simulink library "Renault_Library"  (Simulink 8.2) (continued)
== system Renault_Library/Services ECU/Diag == (continued)
  RSA_DiagnosticManagement_v02.00 : Subsystem  Tag=[LIB]  mask(Diagnostic){DiagnosticSessionControl=on, DefaultSession=Activated, ProgrammingSession=Activated, ExtendedDiagnosticSession=Activated, SafetySystemDiagnosticSession=Activated, EcuReset=off, EcuResetSession1=off, EcuResetSession2=off, EcuResetSession3=off, EcuResetSession4=off, HardReset=off, HardResetSession1=off, HardResetSession2=off, HardResetSession3=off, HardResetSession4=off, KeyOffReset=off, KeyOffResetSession1=off, KeyOffResetSession2=off, KeyOffResetSession3=off, KeyOffResetSession4=off, SoftReset=off, SoftResetSession1=off, SoftResetSession2=off, SoftResetSession3=off, SoftResetSession4=off, EnableRapidPowerShutDown=off, EnableRapidPowerShutDownSession1=off, EnableRapidPowerShutDownSession2=off, EnableRapidPowerShutDownSession3=off, EnableRapidPowerShutDownSession4=off, DisableRapidPowerShutDown=off, DisableRapidPowerShutDownSession1=off, DisableRapidPowerShutDownSession2=off, DisableRapidPowerShutDownSession3=off, DisableRapidPowerShutDownSession4=off, SecurityAccess=off, SecurityAccessSession1=off, SecurityAccessSession2=off, SecurityAccessSession3=off, SecurityAccessSession4=off, RequestSeed=off, RequestSeedSession1=off, RequestSeedSession2=off, RequestSeedSession3=off, RequestSeedSession4=off, SendKey=off, SendKeySession1=off, SendKeySession2=off, SendKeySession3=off, SendKeySession4=off, RequestSeed3=off, RequestSeed3Session1=off, RequestSeed3Session2=off, RequestSeed3Session3=off, RequestSeed3Session4=off, RequestSeed5=off, RequestSeed5Session1=off, RequestSeed5Session2=off, RequestSeed5Session3=off, RequestSeed5Session4=off, RequestSeed7=off, RequestSeed7Session1=off, RequestSeed7Session2=off, RequestSeed7Session3=off, RequestSeed7Session4=off, RequestSeed41=off, RequestSeed41Session1=off, RequestSeed41Session2=off, RequestSeed41Session3=off, RequestSeed41Session4=off, SendKey4=off, SendKey4Session1=off, SendKey4Session2=off, SendKey4Session3=off, SendKey4Session4=off, SendKey6=off, SendKey6Session1=off, SendKey6Session2=off, SendKey6Session3=off, SendKey6Session4=off, SendKey8=off, SendKey8Session1=off, SendKey8Session2=off, SendKey8Session3=off, SendKey8Session4=off, SendKey42=off, SendKey42Session1=off, SendKey42Session2=off, SendKey42Session3=off, SendKey42Session4=off, CommunicationControl=off, CommunicationControlSession1=off, CommunicationControlSession2=off, CommunicationControlSession3=off, CommunicationControlSession4=off, EnableRxAndTx=off, EnableRxAndTxSession1=off, EnableRxAndTxSession2=off, EnableRxAndTxSession3=off, EnableRxAndTxSession4=off, EnableRxAndDisableTx=off, EnableRxAndDisableTxSession1=off, EnableRxAndDisableTxSession2=off, EnableRxAndDisableTxSession3=off, EnableRxAndDisableTxSession4=off, DisableRxAndEnableTx=off, DisableRxAndEnableTxSession1=off, DisableRxAndEnableTxSession2=off, DisableRxAndEnableTxSession3=off, DisableRxAndEnableTxSession4=off, DisableRxAndTx=off, DisableRxAndTxSession1=off, DisableRxAndTxSession2=off, DisableRxAndTxSession3=off, DisableRxAndTxSession4=off, EnableRxAndDisableTxWithEnhanceAddressInformation=off, EnableRxAndDisableTxWithEnhanceAddressInformationSession1=off, EnableRxAndDisableTxWithEnhanceAddressInformationSession2=off, EnableRxAndDisableTxWithEnhanceAddressInformationSession3=off, EnableRxAndDisableTxWithEnhanceAddressInformationSession4=off, EnableRxAndTxWithEnhanceAddressInformation=off, EnableRxAndTxWithEnhanceAddressInformationSession1=off, EnableRxAndTxWithEnhanceAddressInformationSession2=off, EnableRxAndTxWithEnhanceAddressInformationSession3=off, EnableRxAndTxWithEnhanceAddressInformationSession4=off, TesterPresent=on, TesterPresentSession1=on, TesterPresentSession2=on, TesterPresentSession3=on, TesterPresentSession4=on, ZeroSubFunction=on, ZeroSubFunctionSession1=on, ZeroSubFunctionSession2=on, ZeroSubFunctionSession3=on, ZeroSubFunctionSession4=on, ZeroSubFunctionWithoutResponse=on, ZeroSubFunctionWithoutResponseSession1=on, ZeroSubFunctionWithoutResponseSession2=on, ZeroSubFunctionWithoutResponseSession3=on, ZeroSubFunctionWithoutResponseSession4=on, AccessTimingParameter=off, AccessTimingParameterSession1=off, AccessTimingParameterSession2=off, AccessTimingParameterSession3=off, AccessTimingParameterSession4=off, ReadExtendedTimingParamaterSet=off, ReadExtendedTimingParamaterSetSession1=off, ReadExtendedTimingParamaterSetSession2=off, ReadExtendedTimingParamaterSetSession3=off, ReadExtendedTimingParamaterSetSession4=off, SetTimmingParametersToDefaultValues=off, SetTimmingParametersToDefaultValuesSession1=off, SetTimmingParametersToDefaultValuesSession2=off, SetTimmingParametersToDefaultValuesSession3=off, SetTimmingParametersToDefaultValuesSession4=off, ReadCurrentActiveTimmingParamaters=off, ReadCurrentActiveTimmingParamatersSession1=off, ReadCurrentActiveTimmingParamatersSession2=off, ReadCurrentActiveTimmingParamatersSession3=off, ReadCurrentActiveTimmingParamatersSession4=off, SetTimmingParametersToGivenValues=off, SetTimmingParametersToGivenValuesSession1=off, SetTimmingParametersToGivenValuesSession2=off, SetTimmingParametersToGivenValuesSession3=off, SetTimmingParametersToGivenValuesSession4=off, SecuredDataTransmission=off, SecuredDataTransmissionSession1=off, SecuredDataTransmissionSession2=off, SecuredDataTransmissionSession3=off, SecuredDataTransmissionSession4=off, ControlDTCSetting=off, ControlDTCSettingSession1=off, ControlDTCSettingSession2=off, ControlDTCSettingSession3=off, ControlDTCSettingSession4=off, On=off, OnSession1=off, OnSession2=off, OnSession3=off, OnSession4=off, Off=off, OffSession1=off, OffSession2=off, OffSession3=off, OffSession4=off, ResponseOnEvent=off, ResponseOnEventSession1=off, ResponseOnEventSession2=off, ResponseOnEventSession3=off, ResponseOnEventSession4=off, StopResponseOnEvent=off, StopResponseOnEventSession1=off, StopResponseOnEventSession2=off, StopResponseOnEventSession3=off, StopResponseOnEventSession4=off, OnDTCStatusChange=off, OnDTCStatusChangeSession1=off, OnDTCStatusChangeSession2=off, OnDTCStatusChangeSession3=off, OnDTCStatusChangeSession4=off, OnTimeInterrupt=off, OnTimeInterruptSession1=off, OnTimeInterruptSession2=off, OnTimeInterruptSession3=off, OnTimeInterruptSession4=off, OnChangeOfDataIdentifier=off, OnChangeOfDataIdentifierSession1=off, OnChangeOfDataIdentifierSession2=off, OnChangeOfDataIdentifierSession3=off, OnChangeOfDataIdentifierSession4=off, ReportActivedEvents=off, ReportActivedEventsSession1=off, ReportActivedEventsSession2=off, ReportActivedEventsSession3=off, ReportActivedEventsSession4=off, StartResponseOnEvent=off, StartResponseOnEventSession1=off, StartResponseOnEventSession2=off, StartResponseOnEventSession3=off, StartResponseOnEventSession4=off, ClearResponseOnEvent=off, ClearResponseOnEventSession1=off, ClearResponseOnEventSession2=off, ClearResponseOnEventSession3=off, ClearResponseOnEventSession4=off, OnComparisonOfValues=off, OnComparisonOfValuesSession1=off, OnComparisonOfValuesSession2=off, OnComparisonOfValuesSession3=off, OnComparisonOfValuesSession4=off, LinControl=off, LinControlSession1=off, LinControlSession2=off, LinControlSession3=off, LinControlSession4=off, VerifyModeTransitionWithFixedParamater=off, VerifyModeTransitionWithFixedParamaterSession1=off, VerifyModeTransitionWithFixedParamaterSession2=off, VerifyModeTransitionWithFixedParamaterSession3=off, VerifyModeTransitionWithFixedParamaterSession4=off, VerifyModeTransitionWithSpecificParamater=off, VerifyModeTransitionWithSpecificParamaterSession1=off, VerifyModeTransitionWithSpecificParamaterSession2=off, VerifyModeTransitionWithSpecificParamaterSession3=off, VerifyModeTransitionWithSpecificParamaterSession4=off, TransitionMode=off, TransitionModeSession1=off, TransitionModeSession2=off, TransitionModeSession3=off, TransitionModeSession4=off, ReadDataByIdentifier=on, ReadDataByIdentifierSession1=on, ReadDataByIdentifierSession2=on, ReadDataByIdentifierSession3=on, ReadDataByIdentifierSession4=on, ReadMemoryByAddress=on, ReadMemoryByAddressSession1=on, ReadMemoryByAddressSession2=off, ReadMemoryByAddressSession3=off, ReadMemoryByAddressSession4=off, ReadScalingDataByIdentifier=off, ReadScalingDataByIdentifierSession1=off, ReadScalingDataByIdentifierSession2=off, ReadScalingDataByIdentifierSession3=off, ReadScalingDataByIdentifierSession4=off, ReadDataByPeriodicIdentifier=off, ReadDataByPeriodicIdentifierSession1=off, ReadDataByPeriodicIdentifierSession2=off, ReadDataByPeriodicIdentifierSession3=off, ReadDataByPeriodicIdentifierSession4=off, DynamicallyDefineDataIdentifier=off, DynamicallyDefineDataIdentifierSession1=off, DynamicallyDefineDataIdentifierSession2=off, DynamicallyDefineDataIdentifierSession3=off, DynamicallyDefineDataIdentifierSession4=off, DefineByIdentifier=off, DefineByIdentifierSession1=off, DefineByIdentifierSession2=off, DefineByIdentifierSession3=off, DefineByIdentifierSession4=off, DefineByMemoryAddress=off, DefineByMemoryAddressSession1=off, DefineByMemoryAddressSession2=off, DefineByMemoryAddressSession3=off, DefineByMemoryAddressSession4=off, ClearDynamicallyDefinedDataIdentifier=off, ClearDynamicallyDefinedDataIdentifierSession1=off, ClearDynamicallyDefinedDataIdentifierSession2=off, ClearDynamicallyDefinedDataIdentifierSession3=off, ClearDynamicallyDefinedDataIdentifierSession4=off, WriteDataByIdentifier=on, WriteDataByIdentifierSession1=on, WriteDataByIdentifierSession2=on, WriteDataByIdentifierSession3=on, WriteDataByIdentifierSession4=on, WriteDataByAddress=off, WriteDataByAddressSession1=on, WriteDataByAddressSession2=off, WriteDataByAddressSession3=off, WriteDataByAddressSession4=off, ClearDiagnosticInformation=on, ClearDiagnosticInformationSession1=on, ClearDiagnosticInformationSession2=on, ClearDiagnosticInformationSession3=on, ClearDiagnosticInformationSession4=on, ReadDTCInformation=on, ReadDTCInformationSession1=on, ReadDTCInformationSession2=on, ReadDTCInformationSession3=on, ReadDTCInformationSession4=on, ReportNumberOfDTCByStatusMask=on, ReportNumberOfDTCByStatusMaskSession1=on, ReportNumberOfDTCByStatusMaskSession2=on, ReportNumberOfDTCByStatusMaskSession3=on, ReportNumberOfDTCByStatusMaskSession4=on, ReportDTCByStatusMask=on, ReportDTCByStatusMaskSession1=on, ReportDTCByStatusMaskSession2=on, ReportDTCByStatusMaskSession3=on, ReportDTCByStatusMaskSession4=on, ReporDTCSnapshotIdentification=on, ReporDTCSnapshotIdentificationSession1=on, ReporDTCSnapshotIdentificationSession2=on, ReporDTCSnapshotIdentificationSession3=on, ReporDTCSnapshotIdentificationSession4=on, ReporDTCSnapshotRecordByDTCNumber=off, ReporDTCSnapshotRecordByDTCNumberSession1=off, ReporDTCSnapshotRecordByDTCNumberSession2=off, ReporDTCSnapshotRecordByDTCNumberSession3=off, ReporDTCSnapshotRecordByDTCNumberSession4=off, ReportDTCStoredDataByRecordNumber=off, ReportDTCStoredDataByRecordNumberSession1=off, ReportDTCStoredDataByRecordNumberSession2=off, ReportDTCStoredDataByRecordNumberSession3=off, ReportDTCStoredDataByRecordNumberSession4=off, ReportDTCExtendedDataRecordByDTCNumber=off, ReportDTCExtendedDataRecordByDTCNumberSession1=off, ReportDTCExtendedDataRecordByDTCNumberSession2=off, ReportDTCExtendedDataRecordByDTCNumberSession3=off, ReportDTCExtendedDataRecordByDTCNumberSession4=off, ReportNumberOfDTCBySeverityMaskRecord=off, ReportNumberOfDTCBySeverityMaskRecordSession1=off, ReportNumberOfDTCBySeverityMaskRecordSession2=off, ReportNumberOfDTCBySeverityMaskRecordSession3=off, ReportNumberOfDTCBySeverityMaskRecordSession4=off, ReportDTCBySeverityMaskRecord=off, ReportDTCBySeverityMaskRecordSession1=off, ReportDTCBySeverityMaskRecordSession2=off, ReportDTCBySeverityMaskRecordSession3=off, ReportDTCBySeverityMaskRecordSession4=off, ReportSeverityInformationOfDTC=off, ReportSeverityInformationOfDTCSession1=off, ReportSeverityInformationOfDTCSession2=off, ReportSeverityInformationOfDTCSession3=off, ReportSeverityInformationOfDTCSession4=off, ReportSupportedDTC=on, ReportSupportedDTCSession1=on, ReportSupportedDTCSession2=on, ReportSupportedDTCSession3=on, ReportSupportedDTCSession4=on, ReportFirstTestFailedDTC=off, ReportFirstTestFailedDTCSession1=off, ReportFirstTestFailedDTCSession2=off, ReportFirstTestFailedDTCSession3=off, ReportFirstTestFailedDTCSession4=off, ReportFirstConfirmedDTC=off, ReportFirstConfirmedDTCSession1=off, ReportFirstConfirmedDTCSession2=off, ReportFirstConfirmedDTCSession3=off, ReportFirstConfirmedDTCSession4=off, ReportMostRecentTestFailedDTC=off, ReportMostRecentTestFailedDTCSession1=off, ReportMostRecentTestFailedDTCSession2=off, ReportMostRecentTestFailedDTCSession3=off, ReportMostRecentTestFailedDTCSession4=off, ReportMostRecentConfimedDTC=off, ReportMostRecentConfimedDTCSession1=off, ReportMostRecentConfimedDTCSession2=off, ReportMostRecentConfimedDTCSession3=off, ReportMostRecentConfimedDTCSession4=off, ReportMirrorMemoryDTCByStatusMask=off, ReportMirrorMemoryDTCByStatusMaskSession1=off, ReportMirrorMemoryDTCByStatusMaskSession2=off, ReportMirrorMemoryDTCByStatusMaskSession3=off, ReportMirrorMemoryDTCByStatusMaskSession4=off, ReportMirrorMemoryDTCExtendedDataRecordByDTCNumber=off, ReportMirrorMemoryDTCExtendedDataRecordByDTCNumberSession1=off, ReportMirrorMemoryDTCExtendedDataRecordByDTCNumberSession2=off, ReportMirrorMemoryDTCExtendedDataRecordByDTCNumberSession3=off, ReportMirrorMemoryDTCExtendedDataRecordByDTCNumberSession4=off, ReportNumberOfMirrorMemoryDTCByStatusMask=off, ReportNumberOfMirrorMemoryDTCByStatusMaskSession1=off, ReportNumberOfMirrorMemoryDTCByStatusMaskSession2=off, ReportNumberOfMirrorMemoryDTCByStatusMaskSession3=off, ReportNumberOfMirrorMemoryDTCByStatusMaskSession4=off, ReportNumberOfEmissionsRelatedOBDDTCByStatusMask=off, ReportNumberOfEmissionsRelatedOBDDTCByStatusMaskSession1=off, ReportNumberOfEmissionsRelatedOBDDTCByStatusMaskSession2=off, ReportNumberOfEmissionsRelatedOBDDTCByStatusMaskSession3=off, ReportNumberOfEmissionsRelatedOBDDTCByStatusMaskSession4=off, ReportEmissionsRelatedOBDDTCByStatusMask=off, ReportEmissionsRelatedOBDDTCByStatusMaskSession1=off, ReportEmissionsRelatedOBDDTCByStatusMaskSession2=off, ReportEmissionsRelatedOBDDTCByStatusMaskSession3=off, ReportEmissionsRelatedOBDDTCByStatusMaskSession4=off, ReportDTCFaultDetectionCounter=off, ReportDTCFaultDetectionCounterSession1=off, ReportDTCFaultDetectionCounterSession2=off, ReportDTCFaultDetectionCounterSession3=off, ReportDTCFaultDetectionCounterSession4=off, ReportDTCWithPermanetStatus=off, ReportDTCWithPermanetStatusSession1=off, ReportDTCWithPermanetStatusSession2=off, ReportDTCWithPermanetStatusSession3=off, ReportDTCWithPermanetStatusSession4=off, ReportWWHOBDDTCByMaskRecord=off, ReportWWHOBDDTCByMaskRecordSession1=off, ReportWWHOBDDTCByMaskRecordSession2=off, ReportWWHOBDDTCByMaskRecordSession3=off, ReportWWHOBDDTCByMaskRecordSession4=off, ReportWWHOBDDTCWithPermanentStatus=off, ReportWWHOBDDTCWithPermanentStatusSession1=off, ReportWWHOBDDTCWithPermanentStatusSession2=off, ReportWWHOBDDTCWithPermanentStatusSession3=off, ReportWWHOBDDTCWithPermanentStatusSession4=off, InputOutputConrolByIdentifier=on, InputOutputConrolByIdentifierSession1=on, InputOutputConrolByIdentifierSession2=on, InputOutputConrolByIdentifierSession3=on, InputOutputConrolByIdentifierSession4=on, RoutineControl=on, RoutineControlSession1=on, RoutineControlSession2=off, RoutineControlSession3=on, RoutineControlSession4=on, StartRoutine=on, StartRoutineSession1=on, StartRoutineSession2=on, StartRoutineSession3=on, StartRoutineSession4=on, StopRoutine=on, StopRoutineSession1=on, StopRoutineSession2=on, StopRoutineSession3=on, StopRoutineSession4=on, RequestRoutineResults=off, RequestRoutineResultsSession1=off, RequestRoutineResultsSession2=off, RequestRoutineResultsSession3=off, RequestRoutineResultsSession4=off, RequestDownload=off, RequestDownloadSession1=off, RequestDownloadSession2=off, RequestDownloadSession3=off, RequestDownloadSession4=off, RequestUpload=off, RequestUploadSession1=off, RequestUploadSession2=off, RequestUploadSession3=off, RequestUploadSession4=off, TransfertData=off, TransfertDataSession1=off, TransfertDataSession2=off, TransfertDataSession3=off, TransfertDataSession4=off, RequestTransfertExit=off, RequestTransfertExitSession1=off, RequestTransfertExitSession2=off, RequestTransfertExitSession3=off, RequestTransfertExitSession4=off}
      mask prompts: DiagnosticSessionControl "DiagnosticSessionControl (0x10)"; DefaultSession "DefaultSession (0x01)"; ProgrammingSession "ProgrammingSession (0x02)"; ExtendedDiagnosticSession "ExtendedDiagnosticSession (0x03)"; SafetySystemDiagnosticSession "SafetySystemDiagnosticSession (0x04)"; EcuReset "EcuReset (0x11)"; EcuResetSession1 "EcuReset (0x11)"; EcuResetSession2 "EcuReset (0x11)"; EcuResetSession3 "EcuReset (0x11)"; EcuResetSession4 "EcuReset (0x11)"; HardReset "HardReset (0x01)"; HardResetSession1 "HardReset (0x01)"; HardResetSession2 "HardReset (0x01)"; HardResetSession3 "HardReset (0x01)"; HardResetSession4 "HardReset (0x01)"; KeyOffReset "KeyOffReset (0x02)"; KeyOffResetSession1 "KeyOffReset (0x02)"; KeyOffResetSession2 "KeyOffReset (0x02)"; KeyOffResetSession3 "KeyOffReset (0x02)"; KeyOffResetSession4 "KeyOffReset (0x02)"; SoftReset "SoftReset (0x03)"; SoftResetSession1 "SoftReset (0x03)"; SoftResetSession2 "SoftReset (0x03)"; SoftResetSession3 "SoftReset (0x03)"; SoftResetSession4 "SoftReset (0x03)"; EnableRapidPowerShutDown "EnableRapidPowerShutDown (0x04)"; EnableRapidPowerShutDownSession1 "EnableRapidPowerShutDown (0x04)"; EnableRapidPowerShutDownSession2 "EnableRapidPowerShutDown (0x04)"; EnableRapidPowerShutDownSession3 "EnableRapidPowerShutDown (0x04)"; EnableRapidPowerShutDownSession4 "EnableRapidPowerShutDown (0x04)"; DisableRapidPowerShutDown "DisableRapidPowerShutDown (0x05)"; DisableRapidPowerShutDownSession1 "DisableRapidPowerShutDown (0x05)"; DisableRapidPowerShutDownSession2 "DisableRapidPowerShutDown (0x05)"; DisableRapidPowerShutDownSession3 "DisableRapidPowerShutDown (0x05)"; DisableRapidPowerShutDownSession4 "DisableRapidPowerShutDown (0x05)"; SecurityAccess "SecurityAccess (0x27)"; SecurityAccessSession1 "SecurityAccess (0x27)"; SecurityAccessSession2 "SecurityAccess (0x27)"; SecurityAccessSession3 "SecurityAccess (0x27)"; SecurityAccessSession4 "SecurityAccess (0x27)"; RequestSeed "RequestSeed (0x01)"; RequestSeedSession1 "RequestSeed (0x01)"; RequestSeedSession2 "RequestSeed (0x01)"; RequestSeedSession3 "RequestSeed (0x01)"; RequestSeedSession4 "RequestSeed (0x01)"; SendKey "SendKey (0x02)"; SendKeySession1 "SendKey (0x02)"; SendKeySession2 "SendKey (0x02)"; SendKeySession3 "SendKey (0x02)"; SendKeySession4 "SendKey (0x02)"; RequestSeed3 "RequestSeed (0x03)"; RequestSeed3Session1 "RequestSeed (0x03)"; RequestSeed3Session2 "RequestSeed (0x03)"; RequestSeed3Session3 "RequestSeed (0x03)"; RequestSeed3Session4 "RequestSeed (0x03)"; RequestSeed5 "RequestSeed (0x05)"; RequestSeed5Session1 "RequestSeed (0x05)"; RequestSeed5Session2 "RequestSeed (0x05)"; RequestSeed5Session3 "RequestSeed (0x05)"; RequestSeed5Session4 "RequestSeed (0x05)"; RequestSeed7 "RequestSeed (0x07)"; RequestSeed7Session1 "RequestSeed (0x07)"; RequestSeed7Session2 "RequestSeed (0x07)"; RequestSeed7Session3 "RequestSeed (0x07)"; RequestSeed7Session4 "RequestSeed (0x07)"; RequestSeed41 "RequestSeed (0x41)"; RequestSeed41Session1 "RequestSeed (0x41)"; RequestSeed41Session2 "RequestSeed (0x41)"; RequestSeed41Session3 "RequestSeed (0x41)"; RequestSeed41Session4 "RequestSeed (0x41)"; SendKey4 "SendKey (0x04)"; SendKey4Session1 "SendKey (0x04)"; SendKey4Session2 "SendKey (0x04)"; SendKey4Session3 "SendKey (0x04)"; SendKey4Session4 "SendKey (0x04)"; SendKey6 "SendKey (0x06)"; SendKey6Session1 "SendKey (0x06)"; SendKey6Session2 "SendKey (0x06)"; SendKey6Session3 "SendKey (0x06)"; SendKey6Session4 "SendKey (0x06)"; SendKey8 "SendKey (0x08)"; SendKey8Session1 "SendKey (0x08)"; SendKey8Session2 "SendKey (0x08)"; SendKey8Session3 "SendKey (0x08)"; SendKey8Session4 "SendKey (0x08)"; SendKey42 "SendKey (0x42)"; SendKey42Session1 "SendKey (0x42)"; SendKey42Session2 "SendKey (0x42)"; SendKey42Session3 "SendKey (0x42)"; SendKey42Session4 "SendKey (0x42)"; CommunicationControl "CommunicationControl (0x28)"; CommunicationControlSession1 "CommunicationControl (0x28)"; CommunicationControlSession2 "CommunicationControl (0x28)"; CommunicationControlSession3 "CommunicationControl (0x28)"; CommunicationControlSession4 "CommunicationControl (0x28)"; EnableRxAndTx "EnableRxAndTx (0x00)"; EnableRxAndTxSession1 "EnableRxAndTx (0x00)"; EnableRxAndTxSession2 "EnableRxAndTx (0x00)"; EnableRxAndTxSession3 "EnableRxAndTx (0x00)"; EnableRxAndTxSession4 "EnableRxAndTx (0x00)"; EnableRxAndDisableTx "EnableRxAndDisableTx (0x01)"; EnableRxAndDisableTxSession1 "EnableRxAndDisableTx (0x01)"; EnableRxAndDisableTxSession2 "EnableRxAndDisableTx (0x01)"; EnableRxAndDisableTxSession3 "EnableRxAndDisableTx (0x01)"; EnableRxAndDisableTxSession4 "EnableRxAndDisableTx (0x01)"; DisableRxAndEnableTx "DisableRxAndEnableTx (0x02)"; DisableRxAndEnableTxSession1 "DisableRxAndEnableTx (0x02)"; DisableRxAndEnableTxSession2 "DisableRxAndEnableTx (0x02)"; DisableRxAndEnableTxSession3 "DisableRxAndEnableTx (0x02)"; DisableRxAndEnableTxSession4 "DisableRxAndEnableTx (0x02)"; DisableRxAndTx "DisableRxAndTx (0x03)"; DisableRxAndTxSession1 "DisableRxAndTx (0x03)"; DisableRxAndTxSession2 "DisableRxAndTx (0x03)"; DisableRxAndTxSession3 "DisableRxAndTx (0x03)"; DisableRxAndTxSession4 "DisableRxAndTx (0x03)"; EnableRxAndDisableTxWithEnhanceAddressInformation "EnableRxAndDisableTxWithEnhanceAddressInformation (0x04)"; EnableRxAndDisableTxWithEnhanceAddressInformationSession1 "EnableRxAndDisableTxWithEnhanceAddressInformation (0x04)"; EnableRxAndDisableTxWithEnhanceAddressInformationSession2 "EnableRxAndDisableTxWithEnhanceAddressInformation (0x04)"; EnableRxAndDisableTxWithEnhanceAddressInformationSession3 "EnableRxAndDisableTxWithEnhanceAddressInformation (0x04)"; EnableRxAndDisableTxWithEnhanceAddressInformationSession4 "EnableRxAndDisableTxWithEnhanceAddressInformation (0x04)"; EnableRxAndTxWithEnhanceAddressInformation "EnableRxAndTxWithEnhanceAddressInformation (0x05)"; EnableRxAndTxWithEnhanceAddressInformationSession1 "EnableRxAndTxWithEnhanceAddressInformation (0x05)"; EnableRxAndTxWithEnhanceAddressInformationSession2 "EnableRxAndTxWithEnhanceAddressInformation (0x05)"; EnableRxAndTxWithEnhanceAddressInformationSession3 "EnableRxAndTxWithEnhanceAddressInformation (0x05)"; EnableRxAndTxWithEnhanceAddressInformationSession4 "EnableRxAndTxWithEnhanceAddressInformation (0x05)"; TesterPresent "TesterPresent (0x3E)"; TesterPresentSession1 "TesterPresent (0x3E)"; TesterPresentSession2 "TesterPresent (0x3E)"; TesterPresentSession3 "TesterPresent (0x3E)"; TesterPresentSession4 "TesterPresent (0x3E)"; ZeroSubFunction "ZeroSubFunction (0x00)"; ZeroSubFunctionSession1 "ZeroSubFunction (0x00)"; ZeroSubFunctionSession2 "ZeroSubFunction (0x00)"; ZeroSubFunctionSession3 "ZeroSubFunction (0x00)"; ZeroSubFunctionSession4 "ZeroSubFunction (0x00)"; ZeroSubFunctionWithoutResponse "ZeroSubFunctionWithoutResponse (0x80)"; ZeroSubFunctionWithoutResponseSession1 "ZeroSubFunctionWithoutResponse (0x80)"; ZeroSubFunctionWithoutResponseSession2 "ZeroSubFunctionWithoutResponse (0x80)"; ZeroSubFunctionWithoutResponseSession3 "ZeroSubFunctionWithoutResponse (0x80)"; ZeroSubFunctionWithoutResponseSession4 "ZeroSubFunctionWithoutResponse (0x80)"; AccessTimingParameter "AccessTimingParameter (0x83)"; AccessTimingParameterSession1 "AccessTimingParameter (0x83)"; AccessTimingParameterSession2 "AccessTimingParameter (0x83)"; AccessTimingParameterSession3 "AccessTimingParameter (0x83)"; AccessTimingParameterSession4 "AccessTimingParameter (0x83)"; ReadExtendedTimingParamaterSet "ReadExtendedTimingParamaterSet (0x01)"; ReadExtendedTimingParamaterSetSession1 "ReadExtendedTimingParamaterSet (0x01)"; ReadExtendedTimingParamaterSetSession2 "ReadExtendedTimingParamaterSet (0x01)"; ReadExtendedTimingParamaterSetSession3 "ReadExtendedTimingParamaterSet (0x01)"; ReadExtendedTimingParamaterSetSession4 "ReadExtendedTimingParamaterSet (0x01)"; SetTimmingParametersToDefaultValues "SetTimmingParametersToDefaultValues (0x02)"; SetTimmingParametersToDefaultValuesSession1 "SetTimmingParametersToDefaultValues (0x02)"; SetTimmingParametersToDefaultValuesSession2 "SetTimmingParametersToDefaultValues (0x02)"; SetTimmingParametersToDefaultValuesSession3 "SetTimmingParametersToDefaultValues (0x02)"; SetTimmingParametersToDefaultValuesSession4 "SetTimmingParametersToDefaultValues (0x02)"; ReadCurrentActiveTimmingParamaters "ReadCurrentActiveTimmingParamaters (0x03)"; ReadCurrentActiveTimmingParamatersSession1 "ReadCurrentActiveTimmingParamaters (0x03)"; ReadCurrentActiveTimmingParamatersSession2 "ReadCurrentActiveTimmingParamaters (0x03)"; ReadCurrentActiveTimmingParamatersSession3 "ReadCurrentActiveTimmingParamaters (0x03)"; ReadCurrentActiveTimmingParamatersSession4 "ReadCurrentActiveTimmingParamaters (0x03)"; SetTimmingParametersToGivenValues "SetTimmingParametersToGivenValues (0x04)"; SetTimmingParametersToGivenValuesSession1 "SetTimmingParametersToGivenValues (0x04)"; SetTimmingParametersToGivenValuesSession2 "SetTimmingParametersToGivenValues (0x04)"; SetTimmingParametersToGivenValuesSession3 "SetTimmingParametersToGivenValues (0x04)"; SetTimmingParametersToGivenValuesSession4 "SetTimmingParametersToGivenValues (0x04)"; SecuredDataTransmission "SecuredDataTransmission (0x84)"; SecuredDataTransmissionSession1 "SecuredDataTransmission (0x84)"; SecuredDataTransmissionSession2 "SecuredDataTransmission (0x84)"; SecuredDataTransmissionSession3 "SecuredDataTransmission (0x84)"; SecuredDataTransmissionSession4 "SecuredDataTransmission (0x84)"; ControlDTCSetting "ControlDTCSetting (0x85)"; ControlDTCSettingSession1 "ControlDTCSetting (0x85)"; ControlDTCSettingSession2 "ControlDTCSetting (0x85)"; ControlDTCSettingSession3 "ControlDTCSetting (0x85)"; ControlDTCSettingSession4 "ControlDTCSetting (0x85)"; On "On (0x01)"; OnSession1 "On (0x01)"; OnSession2 "On (0x01)"; OnSession3 "On (0x01)"; OnSession4 "On (0x01)"; Off "Off (0x02)"; OffSession1 "Off (0x02)"; OffSession2 "Off (0x02)"; OffSession3 "Off (0x02)"; OffSession4 "Off (0x02)"; ResponseOnEvent "ResponseOnEvent (0x86)"; ResponseOnEventSession1 "ResponseOnEvent (0x86)"; ResponseOnEventSession2 "ResponseOnEvent (0x86)"; ResponseOnEventSession3 "ResponseOnEvent (0x86)"; ResponseOnEventSession4 "ResponseOnEvent (0x86)"; StopResponseOnEvent "StopResponseOnEvent (0x00)"; StopResponseOnEventSession1 "StopResponseOnEvent (0x00)"; StopResponseOnEventSession2 "StopResponseOnEvent (0x00)"; StopResponseOnEventSession3 "StopResponseOnEvent (0x00)"; StopResponseOnEventSession4 "StopResponseOnEvent (0x00)"; OnDTCStatusChange "OnDTCStatusChange (0x01)"; OnDTCStatusChangeSession1 "OnDTCStatusChange (0x01)"; OnDTCStatusChangeSession2 "OnDTCStatusChange (0x01)"; OnDTCStatusChangeSession3 "OnDTCStatusChange (0x01)"; OnDTCStatusChangeSession4 "OnDTCStatusChange (0x01)"; OnTimeInterrupt "OnTimeInterrupt (0x02)"; OnTimeInterruptSession1 "OnTimeInterrupt (0x02)"; OnTimeInterruptSession2 "OnTimeInterrupt (0x02)"; OnTimeInterruptSession3 "OnTimeInterrupt (0x02)"; OnTimeInterruptSession4 "OnTimeInterrupt (0x02)"; OnChangeOfDataIdentifier "OnChangeOfDataIdentifier (0x03)"; OnChangeOfDataIdentifierSession1 "OnChangeOfDataIdentifier (0x03)"; OnChangeOfDataIdentifierSession2 "OnChangeOfDataIdentifier (0x03)"; OnChangeOfDataIdentifierSession3 "OnChangeOfDataIdentifier (0x03)"; OnChangeOfDataIdentifierSession4 "OnChangeOfDataIdentifier (0x03)"; ReportActivedEvents "ReportActivedEvents (0x04)"; ReportActivedEventsSession1 "ReportActivedEvents (0x04)"; ReportActivedEventsSession2 "ReportActivedEvents (0x04)"; ReportActivedEventsSession3 "ReportActivedEvents (0x04)"; ReportActivedEventsSession4 "ReportActivedEvents (0x04)"; StartResponseOnEvent "StartResponseOnEvent (0x05)"; StartResponseOnEventSession1 "StartResponseOnEvent (0x05)"; StartResponseOnEventSession2 "StartResponseOnEvent (0x05)"; StartResponseOnEventSession3 "StartResponseOnEvent (0x05)"; StartResponseOnEventSession4 "StartResponseOnEvent (0x05)"; ClearResponseOnEvent "ClearResponseOnEvent (0x06)"; ClearResponseOnEventSession1 "ClearResponseOnEvent (0x06)"; ClearResponseOnEventSession2 "ClearResponseOnEvent (0x06)"; ClearResponseOnEventSession3 "ClearResponseOnEvent (0x06)"; ClearResponseOnEventSession4 "ClearResponseOnEvent (0x06)"; OnComparisonOfValues "OnComparisonOfValues (0x07)"; OnComparisonOfValuesSession1 "OnComparisonOfValues (0x07)"; OnComparisonOfValuesSession2 "OnComparisonOfValues (0x07)"; OnComparisonOfValuesSession3 "OnComparisonOfValues (0x07)"; OnComparisonOfValuesSession4 "OnComparisonOfValues (0x07)"; LinControl "LinControl (0x87)"; LinControlSession1 "LinControl (0x87)"; LinControlSession2 "LinControl (0x87)"; LinControlSession3 "LinControl (0x87)"; LinControlSession4 "LinControl (0x87)"; VerifyModeTransitionWithFixedParamater "VerifyModeTransitionWithFixedParamater (0x01)"; VerifyModeTransitionWithFixedParamaterSession1 "VerifyModeTransitionWithFixedParamater (0x01)"; VerifyModeTransitionWithFixedParamaterSession2 "VerifyModeTransitionWithFixedParamater (0x01)"; VerifyModeTransitionWithFixedParamaterSession3 "VerifyModeTransitionWithFixedParamater (0x01)"; VerifyModeTransitionWithFixedParamaterSession4 "VerifyModeTransitionWithFixedParamater (0x01)"; VerifyModeTransitionWithSpecificParamater "VerifyModeTransitionWithSpecificParamater (0x02)"; VerifyModeTransitionWithSpecificParamaterSession1 "VerifyModeTransitionWithSpecificParamater (0x02)"; VerifyModeTransitionWithSpecificParamaterSession2 "VerifyModeTransitionWithSpecificParamater (0x02)"; VerifyModeTransitionWithSpecificParamaterSession3 "VerifyModeTransitionWithSpecificParamater (0x02)"; VerifyModeTransitionWithSpecificParamaterSession4 "VerifyModeTransitionWithSpecificParamater (0x02)"; TransitionMode "TransitionMode (0x03)"; TransitionModeSession1 "TransitionMode (0x03)"; TransitionModeSession2 "TransitionMode (0x03)"; TransitionModeSession3 "TransitionMode (0x03)"; TransitionModeSession4 "TransitionMode (0x03)"; ReadDataByIdentifier "ReadDataByIdentifier (0x22)"; ReadDataByIdentifierSession1 "ReadDataByIdentifier (0x22)"; ReadDataByIdentifierSession2 "ReadDataByIdentifier (0x22)"; ReadDataByIdentifierSession3 "ReadDataByIdentifier (0x22)"; ReadDataByIdentifierSession4 "ReadDataByIdentifier (0x22))"; ReadMemoryByAddress "ReadMemoryByAddress (0x23)"; ReadMemoryByAddressSession1 "ReadMemoryByAddress (0x23)"; ReadMemoryByAddressSession2 "ReadMemoryByAddress (0x23)"; ReadMemoryByAddressSession3 "ReadMemoryByAddress (0x23)"; ReadMemoryByAddressSession4 "ReadMemoryByAddress (0x23)"; ReadScalingDataByIdentifier "ReadScalingDataByIdentifier (0x24)"; ReadScalingDataByIdentifierSession1 "ReadScalingDataByIdentifier (0x24)"; ReadScalingDataByIdentifierSession2 "ReadScalingDataByIdentifier (0x24)"; ReadScalingDataByIdentifierSession3 "ReadScalingDataByIdentifier (0x24)"; ReadScalingDataByIdentifierSession4 "ReadScalingDataByIdentifier (0x24)"; ReadDataByPeriodicIdentifier "ReadDataByPeriodicIdentifier (0x2A)"; ReadDataByPeriodicIdentifierSession1 "ReadDataByPeriodicIdentifier (0x2A)"; ReadDataByPeriodicIdentifierSession2 "ReadDataByPeriodicIdentifier (0x2A)"; ReadDataByPeriodicIdentifierSession3 "ReadDataByPeriodicIdentifier (0x2A)"; ReadDataByPeriodicIdentifierSession4 "ReadDataByPeriodicIdentifier (0x2A)"; DynamicallyDefineDataIdentifier "DynamicallyDefineDataIdentifier (0x2C)"; DynamicallyDefineDataIdentifierSession1 "DynamicallyDefineDataIdentifier (0x2C)"; DynamicallyDefineDataIdentifierSession2 "DynamicallyDefineDataIdentifier (0x2C)"; DynamicallyDefineDataIdentifierSession3 "DynamicallyDefineDataIdentifier (0x2C)"; DynamicallyDefineDataIdentifierSession4 "DynamicallyDefineDataIdentifier (0x2C)"; DefineByIdentifier "DefineByIdentifier (0x01)"; DefineByIdentifierSession1 "DefineByIdentifier (0x01)"; DefineByIdentifierSession2 "DefineByIdentifier (0x01)"; DefineByIdentifierSession3 "DefineByIdentifier (0x01)"; DefineByIdentifierSession4 "DefineByIdentifier (0x01)"; DefineByMemoryAddress "DefineByMemoryAddress (0x02)"; DefineByMemoryAddressSession1 "DefineByMemoryAddress (0x02)"; DefineByMemoryAddressSession2 "DefineByMemoryAddress (0x02)"; DefineByMemoryAddressSession3 "DefineByMemoryAddress (0x02)"; DefineByMemoryAddressSession4 "DefineByMemoryAddress (0x02)"; ClearDynamicallyDefinedDataIdentifier "ClearDynamicallyDefinedDataIdentifier (0x03)"; ClearDynamicallyDefinedDataIdentifierSession1 "ClearDynamicallyDefinedDataIdentifier (0x03)"; ClearDynamicallyDefinedDataIdentifierSession2 "ClearDynamicallyDefinedDataIdentifier (0x03)"; ClearDynamicallyDefinedDataIdentifierSession3 "ClearDynamicallyDefinedDataIdentifier (0x03)"; ClearDynamicallyDefinedDataIdentifierSession4 "ClearDynamicallyDefinedDataIdentifier (0x03)"; WriteDataByIdentifier "WriteDataByIdentifier (0x2E)"; WriteDataByIdentifierSession1 "WriteDataByIdentifier (0x2E)"; WriteDataByIdentifierSession2 "WriteDataByIdentifier (0x2E)"; WriteDataByIdentifierSession3 "WriteDataByIdentifier (0x2E)"; WriteDataByIdentifierSession4 "WriteDataByIdentifier (0x2E)"; WriteDataByAddress "WriteDataByAddress (0x3D)"; WriteDataByAddressSession1 "WriteDataByAddress (0x3D)"; WriteDataByAddressSession2 "WriteDataByAddress (0x3D)"; WriteDataByAddressSession3 "WriteDataByAddress (0x3D)"; WriteDataByAddressSession4 "WriteDataByAddress (0x3D)"; ClearDiagnosticInformation "ClearDiagnosticInformation (0x14)"; ClearDiagnosticInformationSession1 "ClearDiagnosticInformation (0x14)"; ClearDiagnosticInformationSession2 "ClearDiagnosticInformation (0x14)"; ClearDiagnosticInformationSession3 "ClearDiagnosticInformation (0x14)"; ClearDiagnosticInformationSession4 "ClearDiagnosticInformation (0x14)"; ReadDTCInformation "ReadDTCInformation (0x19)"; ReadDTCInformationSession1 "ReadDTCInformation (0x19)"; ReadDTCInformationSession2 "ReadDTCInformation (0x19)"; ReadDTCInformationSession3 "ReadDTCInformation (0x19)"; ReadDTCInformationSession4 "ReadDTCInformation (0x19)"; ReportNumberOfDTCByStatusMask "ReportNumberOfDTCByStatusMask (0x01)"; ReportNumberOfDTCByStatusMaskSession1 "ReportNumberOfDTCByStatusMask (0x01)"; ReportNumberOfDTCByStatusMaskSession2 "ReportNumberOfDTCByStatusMask (0x01)"; ReportNumberOfDTCByStatusMaskSession3 "ReportNumberOfDTCByStatusMask (0x01)"; ReportNumberOfDTCByStatusMaskSession4 "ReportNumberOfDTCByStatusMask (0x01)"; ReportDTCByStatusMask "ReportDTCByStatusMask (0x02)"; ReportDTCByStatusMaskSession1 "ReportDTCByStatusMask (0x02)"; ReportDTCByStatusMaskSession2 "ReportDTCByStatusMask (0x02)"; ReportDTCByStatusMaskSession3 "ReportDTCByStatusMask (0x02)"; ReportDTCByStatusMaskSession4 "ReportDTCByStatusMask (0x02)"; ReporDTCSnapshotIdentification "ReporDTCSnapshotIdentification (0x03)"; ReporDTCSnapshotIdentificationSession1 "ReporDTCSnapshotIdentification (0x03)"; ReporDTCSnapshotIdentificationSession2 "ReporDTCSnapshotIdentification (0x03)"; ReporDTCSnapshotIdentificationSession3 "ReporDTCSnapshotIdentification (0x03)"; ReporDTCSnapshotIdentificationSession4 "ReporDTCSnapshotIdentification (0x03)"; ReporDTCSnapshotRecordByDTCNumber "ReporDTCSnapshotRecordByDTCNumber (0x04)"; ReporDTCSnapshotRecordByDTCNumberSession1 "ReporDTCSnapshotRecordByDTCNumber (0x04)"; ReporDTCSnapshotRecordByDTCNumberSession2 "ReporDTCSnapshotRecordByDTCNumber (0x04)"; ReporDTCSnapshotRecordByDTCNumberSession3 "ReporDTCSnapshotRecordByDTCNumber (0x04)"; ReporDTCSnapshotRecordByDTCNumberSession4 "ReporDTCSnapshotRecordByDTCNumber (0x04)"; ReportDTCStoredDataByRecordNumber "ReportDTCStoredDataByRecordNumber (0x05)"; ReportDTCStoredDataByRecordNumberSession1 "ReportDTCStoredDataByRecordNumber (0x05)"; ReportDTCStoredDataByRecordNumberSession2 "ReportDTCStoredDataByRecordNumber (0x05)"; ReportDTCStoredDataByRecordNumberSession3 "ReportDTCStoredDataByRecordNumber (0x05)"; ReportDTCStoredDataByRecordNumberSession4 "ReportDTCStoredDataByRecordNumber (0x05)"; ReportDTCExtendedDataRecordByDTCNumber "ReportDTCExtendedDataRecordByDTCNumber (0x06)"; ReportDTCExtendedDataRecordByDTCNumberSession1 "ReportDTCExtendedDataRecordByDTCNumber (0x06)"; ReportDTCExtendedDataRecordByDTCNumberSession2 "ReportDTCExtendedDataRecordByDTCNumber (0x06)"; ReportDTCExtendedDataRecordByDTCNumberSession3 "ReportDTCExtendedDataRecordByDTCNumber (0x06)"; ReportDTCExtendedDataRecordByDTCNumberSession4 "ReportDTCExtendedDataRecordByDTCNumber (0x06)"; ReportNumberOfDTCBySeverityMaskRecord "ReportNumberOfDTCBySeverityMaskRecord (0x07)"; ReportNumberOfDTCBySeverityMaskRecordSession1 "ReportNumberOfDTCBySeverityMaskRecord (0x07)"; ReportNumberOfDTCBySeverityMaskRecordSession2 "ReportNumberOfDTCBySeverityMaskRecord (0x07)"; ReportNumberOfDTCBySeverityMaskRecordSession3 "ReportNumberOfDTCBySeverityMaskRecord (0x07)"; ReportNumberOfDTCBySeverityMaskRecordSession4 "ReportNumberOfDTCBySeverityMaskRecord (0x07)"; ReportDTCBySeverityMaskRecord "ReportDTCBySeverityMaskRecord (0x08)"; ReportDTCBySeverityMaskRecordSession1 "ReportDTCBySeverityMaskRecord (0x08)"; ReportDTCBySeverityMaskRecordSession2 "ReportDTCBySeverityMaskRecord (0x08)"; ReportDTCBySeverityMaskRecordSession3 "ReportDTCBySeverityMaskRecord (0x08)"; ReportDTCBySeverityMaskRecordSession4 "ReportDTCBySeverityMaskRecord (0x08)"; ReportSeverityInformationOfDTC "ReportSeverityInformationOfDTC (0x09)"; ReportSeverityInformationOfDTCSession1 "ReportSeverityInformationOfDTC (0x09)"; ReportSeverityInformationOfDTCSession2 "ReportSeverityInformationOfDTC (0x09)"; ReportSeverityInformationOfDTCSession3 "ReportSeverityInformationOfDTC (0x09)"; ReportSeverityInformationOfDTCSession4 "ReportSeverityInformationOfDTC (0x09)"; ReportSupportedDTC "ReportSupportedDTC (0x0A)"; ReportSupportedDTCSession1 "ReportSupportedDTC (0x0A)"; ReportSupportedDTCSession2 "ReportSupportedDTC (0x0A)"; ReportSupportedDTCSession3 "ReportSupportedDTC (0x0A)"; ReportSupportedDTCSession4 "ReportSupportedDTC (0x0A)"; ReportFirstTestFailedDTC "ReportFirstTestFailedDTC (0x0B)"; ReportFirstTestFailedDTCSession1 "ReportFirstTestFailedDTC (0x0B)"; ReportFirstTestFailedDTCSession2 "ReportFirstTestFailedDTC (0x0B)"; ReportFirstTestFailedDTCSession3 "ReportFirstTestFailedDTC (0x0B)"; ReportFirstTestFailedDTCSession4 "ReportFirstTestFailedDTC (0x0B)"; ReportFirstConfirmedDTC "ReportFirstConfirmedDTC (0x0C)"; ReportFirstConfirmedDTCSession1 "ReportFirstConfirmedDTC (0x0C)"; ReportFirstConfirmedDTCSession2 "ReportFirstConfirmedDTC (0x0C)"; ReportFirstConfirmedDTCSession3 "ReportFirstConfirmedDTC (0x0C)"; ReportFirstConfirmedDTCSession4 "ReportFirstConfirmedDTC (0x0C)"; ReportMostRecentTestFailedDTC "ReportMostRecentTestFailedDTC (0x0D)"; ReportMostRecentTestFailedDTCSession1 "ReportMostRecentTestFailedDTC (0x0D)"; ReportMostRecentTestFailedDTCSession2 "ReportMostRecentTestFailedDTC (0x0D)"; ReportMostRecentTestFailedDTCSession3 "ReportMostRecentTestFailedDTC (0x0D)"; ReportMostRecentTestFailedDTCSession4 "ReportMostRecentTestFailedDTC (0x0D)"; ReportMostRecentConfimedDTC "ReportMostRecentConfimedDTC (0x0E)"; ReportMostRecentConfimedDTCSession1 "ReportMostRecentConfimedDTC (0x0E))"; ReportMostRecentConfimedDTCSession2 "ReportMostRecentConfimedDTC (0x0E)"; ReportMostRecentConfimedDTCSession3 "ReportMostRecentConfimedDTC (0x0E)"; ReportMostRecentConfimedDTCSession4 "ReportMostRecentConfimedDTC (0x0E)"; ReportMirrorMemoryDTCByStatusMask "ReportMirrorMemoryDTCByStatusMask (0x0F)"; ReportMirrorMemoryDTCByStatusMaskSession1 "ReportMirrorMemoryDTCByStatusMask (0x0F)"; ReportMirrorMemoryDTCByStatusMaskSession2 "ReportMirrorMemoryDTCByStatusMask (0x0F)"; ReportMirrorMemoryDTCByStatusMaskSession3 "ReportMirrorMemoryDTCByStatusMask (0x0F)"; ReportMirrorMemoryDTCByStatusMaskSession4 "ReportMirrorMemoryDTCByStatusMask (0x0F)"; ReportMirrorMemoryDTCExtendedDataRecordByDTCNumber "ReportMirrorMemoryDTCExtendedDataRecordByDTCNumber (0x10)"; ReportMirrorMemoryDTCExtendedDataRecordByDTCNumberSession1 "ReportMirrorMemoryDTCExtendedDataRecordByDTCNumber (0x10)"; ReportMirrorMemoryDTCExtendedDataRecordByDTCNumberSession2 "ReportMirrorMemoryDTCExtendedDataRecordByDTCNumber (0x10)"; ReportMirrorMemoryDTCExtendedDataRecordByDTCNumberSession3 "ReportMirrorMemoryDTCExtendedDataRecordByDTCNumber (0x10)"; ReportMirrorMemoryDTCExtendedDataRecordByDTCNumberSession4 "ReportMirrorMemoryDTCExtendedDataRecordByDTCNumber (0x10)"; ReportNumberOfMirrorMemoryDTCByStatusMask "ReportNumberOfMirrorMemoryDTCByStatusMask (0x11)"; ReportNumberOfMirrorMemoryDTCByStatusMaskSession1 "ReportNumberOfMirrorMemoryDTCByStatusMask (0x11)"; ReportNumberOfMirrorMemoryDTCByStatusMaskSession2 "ReportNumberOfMirrorMemoryDTCByStatusMask (0x11)"; ReportNumberOfMirrorMemoryDTCByStatusMaskSession3 "ReportNumberOfMirrorMemoryDTCByStatusMask (0x11)"; ReportNumberOfMirrorMemoryDTCByStatusMaskSession4 "ReportNumberOfMirrorMemoryDTCByStatusMask (0x11)"; ReportNumberOfEmissionsRelatedOBDDTCByStatusMask "ReportNumberOfEmissionsRelatedOBDDTCByStatusMask (0x12)"; ReportNumberOfEmissionsRelatedOBDDTCByStatusMaskSession1 "ReportNumberOfEmissionsRelatedOBDDTCByStatusMask (0x12)"; ReportNumberOfEmissionsRelatedOBDDTCByStatusMaskSession2 "ReportNumberOfEmissionsRelatedOBDDTCByStatusMask (0x12)"; ReportNumberOfEmissionsRelatedOBDDTCByStatusMaskSession3 "ReportNumberOfEmissionsRelatedOBDDTCByStatusMask (0x12)"; ReportNumberOfEmissionsRelatedOBDDTCByStatusMaskSession4 "ReportNumberOfEmissionsRelatedOBDDTCByStatusMask (0x12)"; ReportEmissionsRelatedOBDDTCByStatusMask "ReportEmissionsRelatedOBDDTCByStatusMask (0x13)"; ReportEmissionsRelatedOBDDTCByStatusMaskSession1 "ReportEmissionsRelatedOBDDTCByStatusMask (0x13)"; ReportEmissionsRelatedOBDDTCByStatusMaskSession2 "ReportEmissionsRelatedOBDDTCByStatusMask (0x13)v"; ReportEmissionsRelatedOBDDTCByStatusMaskSession3 "ReportEmissionsRelatedOBDDTCByStatusMask (0x13)"; ReportEmissionsRelatedOBDDTCByStatusMaskSession4 "ReportEmissionsRelatedOBDDTCByStatusMask (0x13)"; ReportDTCFaultDetectionCounter "ReportDTCFaultDetectionCounter (0x14)"; ReportDTCFaultDetectionCounterSession1 "ReportDTCFaultDetectionCounter (0x14)"; ReportDTCFaultDetectionCounterSession2 "ReportDTCFaultDetectionCounter (0x14)"; ReportDTCFaultDetectionCounterSession3 "ReportDTCFaultDetectionCounter (0x14)"; ReportDTCFaultDetectionCounterSession4 "ReportDTCFaultDetectionCounter (0x14)"; ReportDTCWithPermanetStatus "ReportDTCWithPermanetStatus (0x15)"; ReportDTCWithPermanetStatusSession1 "ReportDTCWithPermanetStatus (0x15)"; ReportDTCWithPermanetStatusSession2 "ReportDTCWithPermanetStatus (0x15)"; ReportDTCWithPermanetStatusSession3 "ReportDTCWithPermanetStatus (0x15)"; ReportDTCWithPermanetStatusSession4 "ReportDTCWithPermanetStatus (0x15)"; ReportWWHOBDDTCByMaskRecord "ReportWWHOBDDTCByMaskRecord (0x42)"; ReportWWHOBDDTCByMaskRecordSession1 "ReportWWHOBDDTCByMaskRecord (0x42)"; ReportWWHOBDDTCByMaskRecordSession2 "ReportWWHOBDDTCByMaskRecord (0x42)"; ReportWWHOBDDTCByMaskRecordSession3 "ReportWWHOBDDTCByMaskRecord (0x42)"; ReportWWHOBDDTCByMaskRecordSession4 "ReportWWHOBDDTCByMaskRecord (0x42)"; ReportWWHOBDDTCWithPermanentStatus "ReportWWHOBDDTCWithPermanentStatus (0x55)"; ReportWWHOBDDTCWithPermanentStatusSession1 "ReportWWHOBDDTCWithPermanentStatus (0x55)"; ReportWWHOBDDTCWithPermanentStatusSession2 "ReportWWHOBDDTCWithPermanentStatus (0x55)"; ReportWWHOBDDTCWithPermanentStatusSession3 "ReportWWHOBDDTCWithPermanentStatus (0x55)"; ReportWWHOBDDTCWithPermanentStatusSession4 "ReportWWHOBDDTCWithPermanentStatus (0x55)"; InputOutputConrolByIdentifier "InputOutputConrolByIdentifier (0x2F)"; InputOutputConrolByIdentifierSession1 "InputOutputConrolByIdentifier (0x2F)"; InputOutputConrolByIdentifierSession2 "InputOutputConrolByIdentifier (0x2F)"; InputOutputConrolByIdentifierSession3 "InputOutputConrolByIdentifier (0x2F)"; InputOutputConrolByIdentifierSession4 "InputOutputConrolByIdentifier (0x2F)"; RoutineControl "RoutineControl (0x31)"; RoutineControlSession1 "RoutineControl (0x31)"; RoutineControlSession2 "RoutineControl (0x31)"; RoutineControlSession3 "RoutineControl (0x31)"; RoutineControlSession4 "RoutineControl (0x31)"; StartRoutine "StartRoutine (0x01)"; StartRoutineSession1 "StartRoutine (0x01)"; StartRoutineSession2 "StartRoutine (0x01)"; StartRoutineSession3 "StartRoutine (0x01)"; StartRoutineSession4 "StartRoutine (0x01)"; StopRoutine "StopRoutine (0x02)"; StopRoutineSession1 "StopRoutine (0x02)"; StopRoutineSession2 "StopRoutine (0x02)"; StopRoutineSession3 "StopRoutine (0x02)"; StopRoutineSession4 "StopRoutine (0x02)"; RequestRoutineResults "RequestRoutineResults (0x03)"; RequestRoutineResultsSession1 "RequestRoutineResults (0x03)"; RequestRoutineResultsSession2 "RequestRoutineResults (0x03)"; RequestRoutineResultsSession3 "RequestRoutineResults (0x03)"; RequestRoutineResultsSession4 "RequestRoutineResults (0x03)"; RequestDownload "RequestDownload (0x34)"; RequestDownloadSession1 "RequestDownload (0x34)"; RequestDownloadSession2 "RequestDownload (0x34)"; RequestDownloadSession3 "RequestDownload (0x34)"; RequestDownloadSession4 "RequestDownload (0x34)"; RequestUpload "RequestUpload (0x35)"; RequestUploadSession1 "RequestUpload (0x35)"; RequestUploadSession2 "RequestUpload (0x35)"; RequestUploadSession3 "RequestUpload (0x35)"; RequestUploadSession4 "RequestUpload (0x35)"; TransfertData "TransfertData (0x36)"; TransfertDataSession1 "TransfertData (0x36)"; TransfertDataSession2 "TransfertData (0x36)"; TransfertDataSession3 "TransfertData (0x36)"; TransfertDataSession4 "TransfertData (0x36)"; RequestTransfertExit "RequestTransfertExit (0x37)"; RequestTransfertExitSession1 "RequestTransfertExit (0x37)"; RequestTransfertExitSession2 "RequestTransfertExit (0x37)"; RequestTransfertExitSession3 "RequestTransfertExit (0x37)"; RequestTransfertExitSession4 "RequestTransfertExit (0x37)"
      mask help:
      | This block performs the diagnostic management.
      |
      | In the library, check the services and sub services supported by the ECU under the tab « Service and SubFunction » and under the tab of each session « Default Session », « Programming Session », « Extented Diagnostic Session » and « Safety System Diagnostic Session », check the services and sub functions supported by each sessions.
      |
      | Version : 02.00
      mask init: same as Renault_Library/Services ECU/Diag/RSA_DiagnosticManagement
      settings: AttributesFormatString=%<Name>
      mask Help:
      | Modification vs 01.00 version
      | 
      | 1) Location : Diagnostic/DIAGNOSTIC_SANCTION_TOP/SessionDiagnostic
      | “Tag visibility” of goto block “EV_TESTER_PRESENT” must be changed from “local” to “global”
      | 
      | 2) Location : Diagnostic/DIAGNOSTIC_ACTION_TOP/Diagnostic_Action
      | The number of “RES_DATA_NUMBER” must be changed from “uint16(1)” to “uint16(2)”
      | 
      | 3) Location : Diagnostic/DIAGNOSTIC_SANCTION_TOP/Sercice19/SubFunction_In_Active_Session_Service19/SubsystemExtenderdSession_19
      | Connection between Constant45 block and RelationOperator138 is missing
      | 
      | 4) Location : Diagnostic/DIAGNOSTIC_SANCTION_TOP/Service2E
      | Adding the constant block set to "True" at the stateflow input
      mask TypeOptions DefaultSession: Activated | Not Activated
      mask TypeOptions ProgrammingSession: Activated | Not Activated
      mask TypeOptions ExtendedDiagnosticSession: Activated | Not Activated
      mask TypeOptions SafetySystemDiagnosticSession: Activated | Not Activated
      mask Display:
      | disp('DIAGNOSTIC\nMANAGEMENT')
      | port_label('input',1,'DTOOL_TO_ECU_RECEIVED')
      | port_label('input',2,'PART1_DTOOL_TO_ECU')
      | port_label('input',3,'PART2_DTOOL_TO_ECU')
      | port_label('input',4,'DIAG_INPUT')
      | port_label('output',1,'ECU_TO_DTOOL_RECEIVED')
      | port_label('output',2,'PART1_ECU_TO_DTOOL')
      | port_label('output',3,'PART2_ECU_TO_DTOOL')
      | port_label('output',4,'DIAG_OUTPUT')
  RSA_FailureManagementSwitch : Subsystem  Tag=[LIB]  mask(RSA_FailureManagementSwitch){}
      mask help:
      | This block can generate faults on the models inputs and outputs.
      | It shall be integrated for each I/O data flow of a Full-ECU.
      |
      | There are 5 type of fault: Output value freeze, Open-loop value application, Short-Circuit to Ground, Short-Circuit to Battery, Open-Circuit
      | If there is no default, the normal value is applied to the output (signal).
      |
      | There are 6 inputs:
      | 	- FunctionalSignal: normal signal
      | 	- Vbat: battery voltage
      | 	- SignalFailure (boolean): default application
      | 	- SignalValue: open loop value
      | 	- SignalApplyValue (boolean): open loop value application
      | 	- SignalFailureType (Enumerated) :  --> 1: no fault
      |                                         --> 2: Freeze
      |                                         --> 3: Value in open-loop
      |                                         --> 4: S.C to Ground
      |                                         --> 5: S.C to battery
      |                                         --> 6: Open-Circuit
      |
      | Version : 01.00
      mask init:
      | GroundValue = 0;
      | OpenCircuitValue = 10^6;
      mask Help:
      | This block can generate faults on the models inputs and outputs.
      | It shall be integrated for each I/O data flow of a Full-ECU.
      | 
      | There are 5 type of fault: Output value freeze, Open-loop value application, Short-Circuit to Ground, Short-Circuit to Battery, Open-Circuit
      | If there is no default, the normal value is applied to the output (signal).
      | 
      | There are 6 inputs:
      | 	- FunctionalSignal: normal signal
      | 	- Vbat: battery voltage
      | 	- SignalFailure (boolean): default application
      | 	- SignalValue: open loop value
      | 	- SignalApplyValue (boolean): open loop value application
      | 	- SignalFailureType (Enumerated) :  --> 1: no fault
      |                                         --> 2: Freeze
      |                                         --> 3: Value in open-loop
      |                                         --> 4: S.C to Ground
      |                                         --> 5: S.C to battery
      |                                         --> 6: Open-Circuit
      | 
      | Version : 01.00
